simulink model "SdbRxSigProc"  (R2022b) (continued)
== system SdbRxSigProc == (continued)
  MemWrForFuTInFuHeatrMdl_MemWrForFuTInFuHeatrMdl : Outport  Port=12  OutDataTypeStr=Bus: MemRecForFuTInFuHeatrMdl  PortDimensions=1  BusOutputAsStruct=on
  MemWrForHeatgInpMgr_MemWrForHeatgInpMgr : Outport  Port=13  OutDataTypeStr=Bus: MemRecForHeatgInpMgr  PortDimensions=1  BusOutputAsStruct=on
  MemWrForSnsrMdlCooltT_MemWrForSnsrMdlCooltT : Outport  Port=14  OutDataTypeStr=Bus: MemRecForSnsrMdlCooltT  PortDimensions=1  BusOutputAsStruct=on
  SunData_SunData : Outport  Port=15  OutDataTypeStr=Bus: SunData  PortDimensions=1  BusOutputAsStruct=on
  SunLoadOutOfVeh_SunLoadOutOfVeh : Outport  Port=16  OutDataTypeStr=Bus: SunLoadOutOfVeh  PortDimensions=1  BusOutputAsStruct=on
  AACAirClngErrStsNgcc_AACAirClngErrStsNgcc : Outport  Port=17  OutDataTypeStr=Enum: AACAirClngErrSts  PortDimensions=1
  AACAirClngStsNgcc_AACAirClngStsNgcc : Outport  Port=18  OutDataTypeStr=Enum: AACAirClngSts  PortDimensions=1
  AFUFragraCh1AvlTiPrecNgcc_AFUFragraCh1AvlTiPrecNgcc : Outport  Port=19  OutMin=0  OutMax=100  OutDataTypeStr=Perc9  PortDimensions=1
  AFUFragraCh2AvlTiPrecNgcc_AFUFragraCh2AvlTiPrecNgcc : Outport  Port=20  OutMin=0  OutMax=100  OutDataTypeStr=Perc9  PortDimensions=1
  AFUFragraCh3AvlTiPrecNgcc_AFUFragraCh3AvlTiPrecNgcc : Outport  Port=21  OutMin=0  OutMax=100  OutDataTypeStr=Perc9  PortDimensions=1
  AFUFragraCh4AvlTiPrecNgcc_AFUFragraCh4AvlTiPrecNgcc : Outport  Port=22  OutMin=0  OutMax=100  OutDataTypeStr=Perc9  PortDimensions=1
  AFUFragraCh5AvlTiPrecNgcc_AFUFragraCh5AvlTiPrecNgcc : Outport  Port=23  OutMin=0  OutMax=100  OutDataTypeStr=Perc9  PortDimensions=1
  AirClngReq_AirClngReq : Outport  Port=24  OutDataTypeStr=Enum: OnOffNoReq  PortDimensions=1
  AirFragCh1OvrdDyNgcc_AirFragCh1OvrdDyNgcc : Outport  Port=25  OutDataTypeStr=Bus: DateTi30  PortDimensions=1  BusOutputAsStruct=on
  AirFragCh2OvrdDyNgcc_AirFragCh2OvrdDyNgcc : Outport  Port=26  OutDataTypeStr=Bus: DateTi30  PortDimensions=1  BusOutputAsStruct=on
  AirFragCh3OvrdDyNgcc_AirFragCh3OvrdDyNgcc : Outport  Port=27  OutDataTypeStr=Bus: DateTi30  PortDimensions=1  BusOutputAsStruct=on
  AirFragCh4OvrdDyNgcc_AirFragCh4OvrdDyNgcc : Outport  Port=28  OutDataTypeStr=Bus: DateTi30  PortDimensions=1  BusOutputAsStruct=on
  AirFragCh5OvrdDyNgcc_AirFragCh5OvrdDyNgcc : Outport  Port=29  OutDataTypeStr=Bus: DateTi30  PortDimensions=1  BusOutputAsStruct=on
  AirPtcEnadNgcc_AirPtcEnadNgcc : Outport  Port=30  OutDataTypeStr=Boolean  PortDimensions=1
  AirPtcHVPwrActlFirstLeNgcc_AirPtcHVPwrActlFirstLeNgcc : Outport  Port=31  OutMin=0  OutMax=20460  OutDataTypeStr=Pwr3  PortDimensions=1
  AirPtcHVPwrActlFirstRiNgcc_AirPtcHVPwrActlFirstRiNgcc : Outport  Port=32  OutMin=0  OutMax=20460  OutDataTypeStr=Pwr3  PortDimensions=1
  AirPtcHVPwrActlSecLeNgcc_AirPtcHVPwrActlSecLeNgcc : Outport  Port=33  OutMin=0  OutMax=20460  OutDataTypeStr=Pwr3  PortDimensions=1
  AirPtcModeReqNgcc_AirPtcModeReqNgcc : Outport  Port=34  OutDataTypeStr=Enum: AirPtcModeReq  PortDimensions=1
  AirSrcStsFbNgcc_AirSrcStsFbNgcc : Outport  Port=35  OutMin=0  OutMax=3  OutDataTypeStr=UInt2  PortDimensions=1
  AltitudeInfromNgcc_AltitudeInfromNgcc : Outport  Port=36  OutDataTypeStr=UInt15  PortDimensions=1
  AMBStsNgcc_AMBStsNgcc : Outport  Port=37  OutDataTypeStr=Bus: AMBStsgrp  PortDimensions=1  BusOutputAsStruct=on
  BackflushVlvErrNgcc_BackflushVlvErrNgcc : Outport  Port=38  OutMin=0  OutMax=3  OutDataTypeStr=UInt2  PortDimensions=1
  BookTrvlClimaActvReq_Ngcc_BookTrvlClimaActvReq : Outport  Port=39  OutDataTypeStr=Enum: OnOffNoReq  PortDimensions=1
  BusCurrentFbNgcc_BusCurrentFbNgcc : Outport  Port=40  OutDataTypeStr=PackCurrent  PortDimensions=1
  ChgFilElmWarnNgcc_ChgFilElmWarnNgcc : Outport  Port=41  OutMin=0  OutMax=3  OutDataTypeStr=UInt2  PortDimensions=1
  CmptmtOxygenConcQFNgcc_CmptmtOxygenConcQFNgcc : Outport  Port=42  OutDataTypeStr=Enum: Qf1  PortDimensions=1
  CmptmtOxygenConcVluNgcc_CmptmtOxygenConcVluNgcc : Outport  Port=43  OutMin=0  OutMax=100  OutDataTypeStr=Perc9  PortDimensions=1
  CoolgFanErrNgcc_CoolgFanErrNgcc : Outport  Port=44  OutMin=0  OutMax=3  OutDataTypeStr=UInt2  PortDimensions=1
  CourseDirFromNavNgcc_CourseDirFromNavNgcc : Outport  Port=45  OutDataTypeStr=Bus: NavDir  PortDimensions=1  BusOutputAsStruct=on
  CurrentCongestionInformationNgcc_CurrentCongestionInformationNgcc : Outport  Port=46  OutMin=0  OutMax=15  OutDataTypeStr=UInt4  PortDimensions=1
  CurrentTunnelInformationNgcc_CurrentTunnelInformationNgcc : Outport  Port=47  OutDataTypeStr=Enum: OnOffNoReq  PortDimensions=1
  DcDcActvdNgcc_DcDcActvdNgcc : Outport  Port=48  OutDataTypeStr=Enum: DcDcActvd  PortDimensions=1
  DcDcPwrLimNgcc_DcDcPwrLimNgcc : Outport  Port=49  OutDataTypeStr=Boolean  PortDimensions=1
  DHUResdSig10Ngcc_DHUResdSig10Ngcc : Outport  Port=50  OutDataTypeStr=UInt32  PortDimensions=1
  DHUResdSig11Ngcc_DHUResdSig11Ngcc : Outport  Port=51  OutDataTypeStr=Unit64  PortDimensions=1
  DHUResdSig12Ngcc_DHUResdSig12Ngcc : Outport  Port=52  OutDataTypeStr=Unit64  PortDimensions=1
  DHUResdSig13Ngcc_DHUResdSig13Ngcc : Outport  Port=53  OutDataTypeStr=UInt32  PortDimensions=1
  DHUResdSig3Ngcc_DHUResdSig3Ngcc : Outport  Port=54  OutDataTypeStr=UInt16  PortDimensions=1
  DHUResdSig4Ngcc_DHUResdSig4Ngcc : Outport  Port=55  OutDataTypeStr=UInt16  PortDimensions=1
  DHUResdSig5Ngcc_DHUResdSig5Ngcc : Outport  Port=56  OutDataTypeStr=UInt32  PortDimensions=1
  DHUResdSig6Ngcc_DHUResdSig6Ngcc : Outport  Port=57  OutDataTypeStr=Unit64  PortDimensions=1
  DHUResdSig7Ngcc_DHUResdSig7Ngcc : Outport  Port=58  OutDataTypeStr=Unit64  PortDimensions=1
  DHUResdSig8Ngcc_DHUResdSig8Ngcc : Outport  Port=59  OutDataTypeStr=UInt16  PortDimensions=1
  DHUResdSig9Ngcc_DHUResdSig9Ngcc : Outport  Port=60  OutDataTypeStr=UInt16  PortDimensions=1
  DispHvBattLvlOfChrgNgcc_DispHvBattLvlOfChrgNgcc : Outport  Port=61  OutMin=0  OutMax=102.30000000000001  OutDataTypeStr=EcoDrvInfo  PortDimensions=1
  DropTopPosnStsNgcc_DropTopPosnStsNgcc : Outport  Port=62  OutDataTypeStr=Enum: PosnSts  PortDimensions=1
  DrvrSeatPosPercNgcc_DrvrSeatPosPercNgcc : Outport  Port=63  OutDataTypeStr=Bus: SeatPosPercQF  PortDimensions=1  BusOutputAsStruct=on
  DrvrSeatSts_Ngcc_DrvrSeatSts : Outport  Port=64  OutDataTypeStr=Enum: OccptPresSt1  PortDimensions=1
  DrvrSeatVentnLvlSts2Ngcc_DrvrSeatVentnLvlSts2Ngcc : Outport  Port=65  OutDataTypeStr=Enum: SeatClimaLvl  PortDimensions=1
  FctvStsNgcc_FctvStsNgcc : Outport  Port=66  OutDataTypeStr=Bus: ValveSts  PortDimensions=1  BusOutputAsStruct=on
  FloatDrvingActvNgcc_FloatDrvingActvNgcc : Outport  Port=67  OutDataTypeStr=Bus: FloatDrvingActv  PortDimensions=1  BusOutputAsStruct=on
  HighTempCleanHmiReqNgcc_HighTempCleanHmiReqNgcc : Outport  Port=68  OutDataTypeStr=Enum: NoReqOnOff  PortDimensions=1
  HMIAirCdnrAutDrySetNgcc_HMIAirCdnrAutDrySetNgcc : Outport  Port=69  OutDataTypeStr=Enum: OnOff1  PortDimensions=1
  HmiAirDistbnRow1LeNgcc_HmiAirDistbnRow1LeNgcc : Outport  Port=70  OutDataTypeStr=Enum: HmiCmptmtAirDistbnFrnt  PortDimensions=1
  HmiAirDistbnRow1RiNgcc_HmiAirDistbnRow1RiNgcc : Outport  Port=71  OutDataTypeStr=Enum: HmiCmptmtAirDistbnFrnt  PortDimensions=1
  HmiAutoRow1LeNgcc_HmiAutoRow1LeNgcc : Outport  Port=72  OutDataTypeStr=Enum: OnOff1  PortDimensions=1
  HmiAutoRow1RiNgcc_HmiAutoRow1RiNgcc : Outport  Port=73  OutDataTypeStr=Enum: OnOff1  PortDimensions=1
  HmiAutOxySetNgcc_HmiAutOxySetNgcc : Outport  Port=74  OutDataTypeStr=Enum: OnOff1  PortDimensions=1
  HmiCarLockACRecircSetNgcc_HmiCarLockACRecircSetNgcc : Outport  Port=75  OutMin=0  OutMax=3  OutDataTypeStr=UInt2  PortDimensions=1
  HmiClimaRow1LePwrNgcc_HmiClimaRow1LePwrNgcc : Outport  Port=76  OutDataTypeStr=Enum: OnOff1  PortDimensions=1
  HmiClimaRow1PwrNgcc_HmiClimaRow1PwrNgcc : Outport  Port=77  OutDataTypeStr=Enum: OnOff1  PortDimensions=1
  HmiClimaRow1RiPwrNgcc_HmiClimaRow1RiPwrNgcc : Outport  Port=78  OutDataTypeStr=Enum: OnOff1  PortDimensions=1
  HmiClimaRow2PwrNgcc_HmiClimaRow2PwrNgcc : Outport  Port=79  OutDataTypeStr=Enum: OnOff1  PortDimensions=1
  HmiClimaRow3PwrNgcc_HmiClimaRow3PwrNgcc : Outport  Port=80  OutDataTypeStr=Enum: OnOff1  PortDimensions=1
  HMIClimaVentSetNgcc_HMIClimaVentSetNgcc : Outport  Port=81  OutDataTypeStr=Enum: OnOff1  PortDimensions=1
  HmiDrvrHeadPosnNgcc_HmiDrvrHeadPosnNgcc : Outport  Port=82  OutDataTypeStr=Bus: GazeDirOri  PortDimensions=1  BusOutputAsStruct=on
  HmiElecAirBodyVentLebtnNgcc_HmiElecAirBodyVentLebtnNgcc : Outport  Port=83  OutMin=0  OutMax=15  OutDataTypeStr=UInt4  PortDimensions=1
  HmiElecAirBodyVentRibtnNgcc_HmiElecAirBodyVentRibtnNgcc : Outport  Port=84  OutMin=0  OutMax=15  OutDataTypeStr=UInt4  PortDimensions=1
  HmiElecAirConsolePosnNgcc_HmiElecAirConsolePosnNgcc : Outport  Port=85  OutDataTypeStr=Bus: PosIndct  PortDimensions=1  BusOutputAsStruct=on
  HmiElecAirConsoleSwtLeNgcc_HmiElecAirConsoleSwtLeNgcc : Outport  Port=86  OutDataTypeStr=Enum: OnOff1  PortDimensions=1
  HmiElecAirConsoleSwtRiNgcc_HmiElecAirConsoleSwtRiNgcc : Outport  Port=87  OutDataTypeStr=Enum: OnOff1  PortDimensions=1
  HmiElecAirDirModeSecRowReqNgcc_HmiElecAirDirModeSecRowReqNgcc : Outport  Port=88  OutDataTypeStr=Bus: DirModReq1  PortDimensions=1  BusOutputAsStruct=on
  HmiElecAirDirSwngSecLeNgcc_HmiElecAirDirSwngSecLeNgcc : Outport  Port=89  OutDataTypeStr=Enum: OnOff1  PortDimensions=1
  HmiElecAirDirSwngSecRiNgcc_HmiElecAirDirSwngSecRiNgcc : Outport  Port=90  OutDataTypeStr=Enum: OnOff1  PortDimensions=1
  HmiElecAirDrvrNatureWindReqNgcc_HmiElecAirDrvrNatureWindReqNgcc : Outport  Port=91  OutDataTypeStr=Enum: OnOff1  PortDimensions=1
  HmiElecAirPassNatureWindReqNgcc_HmiElecAirPassNatureWindReqNgcc : Outport  Port=92  OutDataTypeStr=Enum: OnOff1  PortDimensions=1
  HMIFACSwtNgcc_HMIFACSwtNgcc : Outport  Port=93  OutDataTypeStr=Enum: OnOff1  PortDimensions=1
  HmiFanLvlRow1LeNgcc_HmiFanLvlRow1LeNgcc : Outport  Port=94  OutDataTypeStr=Enum: HmiHvacFanLvl  PortDimensions=1
  HmiFanLvlRow1RiNgcc_HmiFanLvlRow1RiNgcc : Outport  Port=95  OutDataTypeStr=Enum: HmiHvacFanLvl  PortDimensions=1
  HmiFragraChRatReq_HmiFragraChRatReq : Outport  Port=96  OutDataTypeStr=Bus: HmiFragraTypRatReq  PortDimensions=1  BusOutputAsStruct=on
  HmiFragraLvlReq_HmiFragraLvlReq : Outport  Port=97  OutDataTypeStr=Enum: HmiFragraLvlReq  PortDimensions=1
  HMILBRACStrtSetNgcc_HMILBRACStrtSetNgcc : Outport  Port=98  OutDataTypeStr=Enum: OnOff1  PortDimensions=1
  HmiOxyinCarSwtNgcc_HmiOxyinCarSwtNgcc : Outport  Port=99  OutDataTypeStr=Enum: NoReqOnOff  PortDimensions=1
  HmiParkVentSetNgcc_HmiParkVentSetNgcc : Outport  Port=100  OutDataTypeStr=Enum: OnOff1  PortDimensions=1
  HmiParkVentTmrSetNgcc_HmiParkVentTmrSetNgcc : Outport  Port=101  OutMin=0  OutMax=31  OutDataTypeStr=TimeHr5  PortDimensions=1
  HmiPassHeadPosnNgcc_HmiPassHeadPosnNgcc : Outport  Port=102  OutDataTypeStr=Bus: GazeDirOri  PortDimensions=1  BusOutputAsStruct=on
  HmiPopUpResp_HmiPopUpResp : Outport  Port=103  OutDataTypeStr=Enum: BookChargeSetResponse  PortDimensions=1
  HmiSmrtWindFolwSetNgcc_HmiSmrtWindFolwSetNgcc : Outport  Port=104  OutDataTypeStr=Enum: OnOff1  PortDimensions=1
  HMIThermoTankDoorOpenNgcc_HMIThermoTankDoorOpenNgcc : Outport  Port=105  OutDataTypeStr=Enum: OnOffNoReq  PortDimensions=1
  HMIThermoTankEcoSwNgcc_HMIThermoTankEcoSwNgcc : Outport  Port=106  OutDataTypeStr=Enum: NoReqOnOff  PortDimensions=1
  HMIThermoTankTNgcc_HMIThermoTankTNgcc : Outport  Port=107  OutDataTypeStr=TempAtTime  PortDimensions=1
  HmiThermoTSteDeoNewSwtNgcc_HmiThermoTSteDeoNewSwtNgcc : Outport  Port=108  OutDataTypeStr=UInt8  PortDimensions=1
  HmiThermoTSteDeoSwtNgcc_HmiThermoTSteDeoSwtNgcc : Outport  Port=109  OutDataTypeStr=Enum: OnOff1  PortDimensions=1
  HmiUVSterilSetNgcc_HmiUVSterilSetNgcc : Outport  Port=110  OutDataTypeStr=Enum: OnOff1  PortDimensions=1
  HmiWinterLockCarPrevnIceSetNgcc_HmiWinterLockCarPrevnIceSetNgcc : Outport  Port=111  OutDataTypeStr=Enum: OnOff1  PortDimensions=1
  HoodStsNgcc_HoodStsNgcc : Outport  Port=112  OutDataTypeStr=Enum: DoorSts2  PortDimensions=1
  HvBattLimnIndcnNgcc_HvBattLimnIndcnNgcc : Outport  Port=113  OutDataTypeStr=UInt16  PortDimensions=1
  InflateAirSrcSwtNgcc_InflateAirSrcSwtNgcc : Outport  Port=114  OutDataTypeStr=Enum: OnOffNoReq  PortDimensions=1
  InpVoltFbNgcc_InpVoltFbNgcc : Outport  Port=115  OutMin=0  OutMax=1638.3000000000002  OutDataTypeStr=RlyMeaPointVolt  PortDimensions=1
  LiOprnModNgcc_LiOprnModNgcc : Outport  Port=116  OutDataTypeStr=Enum: LiOperMod  PortDimensions=1
  LonglifeBatteryReqNgcc_LonglifeBatteryReqNgcc : Outport  Port=117  OutDataTypeStr=Enum: BatteryReq  PortDimensions=1
  McuOvrTempErrNgcc_McuOvrTempErrNgcc : Outport  Port=118  OutMin=0  OutMax=3  OutDataTypeStr=UInt2  PortDimensions=1
  McuTempFbNgcc_McuTempFbNgcc : Outport  Port=119  OutMin=-256  OutMax=255.9  OutDataTypeStr=MvBattTemp  PortDimensions=1
  McuTempSnsrErrNgcc_McuTempSnsrErrNgcc : Outport  Port=120  OutMin=0  OutMax=3  OutDataTypeStr=UInt2  PortDimensions=1
  MoleculeVlv1Ngcc_MoleculeVlv1Ngcc : Outport  Port=121  OutMin=0  OutMax=3  OutDataTypeStr=UInt2  PortDimensions=1
  MoleculeVlv2Ngcc_MoleculeVlv2Ngcc : Outport  Port=122  OutMin=0  OutMax=3  OutDataTypeStr=UInt2  PortDimensions=1
  MosOvrTempErrNgcc_MosOvrTempErrNgcc : Outport  Port=123  OutMin=0  OutMax=3  OutDataTypeStr=UInt2  PortDimensions=1
  MosTempFbNgcc_MosTempFbNgcc : Outport  Port=124  OutMin=-256  OutMax=255.9  OutDataTypeStr=MvBattTemp  PortDimensions=1
  MosTempSnsrErrNgcc_MosTempSnsrErrNgcc : Outport  Port=125  OutMin=0  OutMax=3  OutDataTypeStr=UInt2  PortDimensions=1
  MotStsFrmOGMNgcc_MotStsFrmOGMNgcc : Outport  Port=126  OutDataTypeStr=UInt8  PortDimensions=1
  MotTempFbNgcc_MotTempFbNgcc : Outport  Port=127  OutMin=-256  OutMax=255.9  OutDataTypeStr=MvBattTemp  PortDimensions=1
  MotTempSnsrErrNgcc_MotTempSnsrErrNgcc : Outport  Port=128  OutMin=0  OutMax=3  OutDataTypeStr=UInt2  PortDimensions=1
  OGMCmprSpdFbNgcc_OGMCmprSpdFbNgcc : Outport  Port=129  OutDataTypeStr=UInt16  PortDimensions=1
  OGMFlowFbNgcc_OGMFlowFbNgcc : Outport  Port=130  OutMin=0  OutMax=51.1  OutDataTypeStr=CooltFlow2  PortDimensions=1
  OGMHwVersNgcc_OGMHwVersNgcc : Outport  Port=131  OutDataTypeStr=UInt16  PortDimensions=1
  OGMIntErrNgcc_OGMIntErrNgcc : Outport  Port=132  OutDataTypeStr=Enum: Err1  PortDimensions=1
  OGMOvrVoltRangErrNgcc_OGMOvrVoltRangErrNgcc : Outport  Port=133  OutDataTypeStr=Enum: Err1  PortDimensions=1
  OGMRunTiNgcc_OGMRunTiNgcc : Outport  Port=134  OutDataTypeStr=UInt32  PortDimensions=1
  OGMSwVersNgcc_OGMSwVersNgcc : Outport  Port=135  OutDataTypeStr=UInt16  PortDimensions=1
  OGMTempErrNgcc_OGMTempErrNgcc : Outport  Port=136  OutDataTypeStr=Enum: TempErr  PortDimensions=1
  OGMUndVoltRangErrNgcc_OGMUndVoltRangErrNgcc : Outport  Port=137  OutDataTypeStr=Enum: Err1  PortDimensions=1
  OxygenConcFbFrmOGMNgcc_OxygenConcFbFrmOGMNgcc : Outport  Port=138  OutMin=0  OutMax=100  OutDataTypeStr=Perc9  PortDimensions=1
  OxygenConcFltNgcc_OxygenConcFltNgcc : Outport  Port=139  OutMin=0  OutMax=3  OutDataTypeStr=UInt2  PortDimensions=1
  OxygenConcSnsrElecErrNgcc_OxygenConcSnsrElecErrNgcc : Outport  Port=140  OutDataTypeStr=Enum: OxygenConcSnsrElecErr  PortDimensions=1
  OxygenConcSnsrIntErrNgcc_OxygenConcSnsrIntErrNgcc : Outport  Port=141  OutDataTypeStr=Enum: Err1  PortDimensions=1
  OxygenConcSnsrOvrVoltRangErrNgcc_OxygenConcSnsrOvrVoltRangErrNgcc : Outport  Port=142  OutDataTypeStr=Enum: Err1  PortDimensions=1
  OxygenConcSnsrStsFrmOGMNgcc_OxygenConcSnsrStsFrmOGMNgcc : Outport  Port=143  OutMin=0  OutMax=3  OutDataTypeStr=UInt2  PortDimensions=1
  OxygenConcSnsrStsNgcc_OxygenConcSnsrStsNgcc : Outport  Port=144  OutDataTypeStr=Enum: PmSnsrSts  PortDimensions=1
  OxygenConcSnsrTempErrNgcc_OxygenConcSnsrTempErrNgcc : Outport  Port=145  OutDataTypeStr=Enum: TempErr  PortDimensions=1
  OxygenConcSnsrUndVoltRangErrNgcc_OxygenConcSnsrUndVoltRangErrNgcc : Outport  Port=146  OutDataTypeStr=Enum: Err1  PortDimensions=1
  OxygenGenrErrInfoNgcc_OxygenGenrErrInfoNgcc : Outport  Port=147  OutDataTypeStr=Enum: OxygengenrErrInfo  PortDimensions=1
  OxygenSplyAvlStsNgcc_OxygenSplyAvlStsNgcc : Outport  Port=148  OutDataTypeStr=Enum: OxygenSplyAvlSts  PortDimensions=1
  OxygenSplyErrNgcc_OxygenSplyErrNgcc : Outport  Port=149  OutDataTypeStr=Enum: Err1  PortDimensions=1
  OxygenTubeBlkNgcc_OxygenTubeBlkNgcc : Outport  Port=150  OutMin=0  OutMax=3  OutDataTypeStr=UInt2  PortDimensions=1
  PassSeatPosPercNgcc_PassSeatPosPercNgcc : Outport  Port=151  OutDataTypeStr=Bus: SeatPosPercQF  PortDimensions=1  BusOutputAsStruct=on
  PassSeatSts2_Ngcc_PassSeatSts2 : Outport  Port=152  OutDataTypeStr=Enum: OccptPresSt1  PortDimensions=1
  PassSeatVentnLvlSts2Ngcc_PassSeatVentnLvlSts2Ngcc : Outport  Port=153  OutDataTypeStr=Enum: SeatClimaLvl  PortDimensions=1
  PhaseCurrentFbNgcc_PhaseCurrentFbNgcc : Outport  Port=154  OutDataTypeStr=PackCurrent  PortDimensions=1
  PosnBriefNgcc_PosnBriefNgcc : Outport  Port=155  OutDataTypeStr=Bus: Posn  PortDimensions=1  BusOutputAsStruct=on
  PressureFbNgcc_PressureFbNgcc : Outport  Port=156  OutMin=0  OutMax=400  OutDataTypeStr=Pressure2  PortDimensions=1
  PressureFltNgcc_PressureFltNgcc : Outport  Port=157  OutMin=0  OutMax=3  OutDataTypeStr=UInt2  PortDimensions=1
  PressureSnsrErrNgcc_PressureSnsrErrNgcc : Outport  Port=158  OutMin=0  OutMax=3  OutDataTypeStr=UInt2  PortDimensions=1
  PwrCnsForHvacHeatrElecRe_PwrCnsForHvacHeatrElecRe : Outport  Port=159  OutDataTypeStr=Pwr33  PortDimensions=1
  RefrLpSwStsNgcc_RefrLpSwStsNgcc : Outport  Port=160  OutDataTypeStr=Enum: CloseOpen  PortDimensions=1
  RefrtorVlvEnaNgcc_RefrtorVlvEnaNgcc : Outport  Port=161  OutDataTypeStr=Enum: OnOff1  PortDimensions=1
  RoadInclnNgcc_RoadInclnNgcc : Outport  Port=162  OutDataTypeStr=Bus: RoadInclnRec1  PortDimensions=1  BusOutputAsStruct=on
  RuranACModeSetNgcc_RuranACModeSetNgcc : Outport  Port=163  OutDataTypeStr=Enum: RuranACModeSet  PortDimensions=1
  SceneModSeld_Ngcc_SceneModSeld : Outport  Port=164  OutDataTypeStr=Enum: PatSeld  PortDimensions=1
  SeatBackAngleRowFirstDrvrNgcc_SeatBackAngleRowFirstDrvrNgcc : Outport  Port=165  OutMin=0  OutMax=180  OutDataTypeStr=AngleDeg180  PortDimensions=1
  SeatBackAngleRowFirstPassNgcc_SeatBackAngleRowFirstPassNgcc : Outport  Port=166  OutMin=0  OutMax=180  OutDataTypeStr=AngleDeg180  PortDimensions=1
  SeatOccptAtRowSecDrSide2_Ngcc_SeatOccptAtRowSecDrSide2 : Outport  Port=167  OutDataTypeStr=Enum: OccptPresSt1  PortDimensions=1
  SeatOccptAtRowSecMid2_Ngcc_SeatOccptAtRowSecMid2 : Outport  Port=168  OutDataTypeStr=Enum: OccptPresSt1  PortDimensions=1
  SeatOccptAtRowSecPasSide2_Ngcc_SeatOccptAtRowSecPasSide2 : Outport  Port=169  OutDataTypeStr=Enum: OccptPresSt1  PortDimensions=1
  SeatOccptAtRowThrdLe2Ngcc_SeatOccptAtRowThrdLe2Ngcc : Outport  Port=170  OutDataTypeStr=Enum: OccptPresSt1  PortDimensions=1
  SeatOccptAtRowThrdRi2Ngcc_SeatOccptAtRowThrdRi2Ngcc : Outport  Port=171  OutDataTypeStr=Enum: OccptPresSt1  PortDimensions=1
  SportModeThmgtRequestNgcc_SportModeThmgtRequestNgcc : Outport  Port=172  OutDataTypeStr=Enum: ThmgtLevel  PortDimensions=1
  TCSCooltFlowNgcc_TCSCooltFlowNgcc : Outport  Port=173  OutDataTypeStr=CooltFlow1  PortDimensions=1
  TCSCooltTNgcc_TCSCooltTNgcc : Outport  Port=174  OutDataTypeStr=TempAtTime  PortDimensions=1
  TcsDoorEleBailReqNgcc_TcsDoorEleBailReqNgcc : Outport  Port=175  OutDataTypeStr=Enum: WhlMotSysCluReq  PortDimensions=1
  TcsFanReqNgcc_TcsFanReqNgcc : Outport  Port=176  OutMin=0  OutMax=15  OutDataTypeStr=UInt4  PortDimensions=1
  TcsModSwtReqNgcc_TcsModSwtReqNgcc : Outport  Port=177  OutDataTypeStr=Enum: TelmThermoTankReq  PortDimensions=1
  TcsOvrheatdProtnStsNgcc_TcsOvrheatdProtnStsNgcc : Outport  Port=178  OutMin=0  OutMax=3  OutDataTypeStr=UInt2  PortDimensions=1
  TcsSemiconReqNgcc_TcsSemiconReqNgcc : Outport  Port=179  OutMin=0  OutMax=15  OutDataTypeStr=UInt4  PortDimensions=1
  TcsTmrOverReqNgcc_TcsTmrOverReqNgcc : Outport  Port=180  OutMin=0  OutMax=31  OutDataTypeStr=TimeHr5  PortDimensions=1
  TcsTNowCtrlReqNgcc_TcsTNowCtrlReqNgcc : Outport  Port=181  OutDataTypeStr=TempAtTime  PortDimensions=1
  TcsTopDoorEleBailReqNgcc_TcsTopDoorEleBailReqNgcc : Outport  Port=182  OutDataTypeStr=Enum: WhlMotSysCluReq  PortDimensions=1
  TelmOxygenValReqNgcc_TelmOxygenValReqNgcc : Outport  Port=183  OutDataTypeStr=Enum: OnOffNoReq  PortDimensions=1
  TelmThermoTankECOSetNgcc_TelmThermoTankECOSetNgcc : Outport  Port=184  OutDataTypeStr=Enum: NoReqOnOff  PortDimensions=1
  TelmThermoTankTNgcc_TelmThermoTankTNgcc : Outport  Port=185  OutDataTypeStr=EngT2  PortDimensions=1
  ThermHvacRecircReqEnaNgcc_ThermHvacRecircReqEnaNgcc : Outport  Port=186  OutDataTypeStr=Boolean  PortDimensions=1
  ThermHvacRecircReqNgcc_ThermHvacRecircReqNgcc : Outport  Port=187  OutMin=0  OutMax=100  OutDataTypeStr=Perc6  PortDimensions=1
  ThrdRowSeatHeatgActlPwrCnsLeNgcc_ThrdRowSeatHeatgActlPwrCnsLeNgcc : Outport  Port=188  OutDataTypeStr=UInt8  PortDimensions=1
  ThrdRowSeatHeatgActlPwrCnsRiNgcc_ThrdRowSeatHeatgActlPwrCnsRiNgcc : Outport  Port=189  OutDataTypeStr=UInt8  PortDimensions=1
  ThrdRowSeatHeatgDesPwrCnsLeNgcc_ThrdRowSeatHeatgDesPwrCnsLeNgcc : Outport  Port=190  OutDataTypeStr=UInt8  PortDimensions=1
  ThrdRowSeatHeatgDesPwrCnsRiNgcc_ThrdRowSeatHeatgDesPwrCnsRiNgcc : Outport  Port=191  OutDataTypeStr=UInt8  PortDimensions=1
  ThrdRowSeatVentnActlPwrCnsLeNgcc_ThrdRowSeatVentnActlPwrCnsLeNgcc : Outport  Port=192  OutDataTypeStr=UInt8  PortDimensions=1
  ThrdRowSeatVentnActlPwrCnsRiNgcc_ThrdRowSeatVentnActlPwrCnsRiNgcc : Outport  Port=193  OutDataTypeStr=UInt8  PortDimensions=1
  ThrdRowSeatVentnDesPwrCnsLeNgcc_ThrdRowSeatVentnDesPwrCnsLeNgcc : Outport  Port=194  OutDataTypeStr=UInt8  PortDimensions=1
  ThrdRowSeatVentnDesPwrCnsRiNgcc_ThrdRowSeatVentnDesPwrCnsRiNgcc : Outport  Port=195  OutDataTypeStr=UInt8  PortDimensions=1
  UseEvapReqTMinNewNgcc_UseEvapReqTMinNewNgcc : Outport  Port=196  OutDataTypeStr=Enum: OnOff1  PortDimensions=1
  VehTiAndDataNgcc_VehTiAndDataNgcc : Outport  Port=197  OutDataTypeStr=Bus: DateTi30  PortDimensions=1  BusOutputAsStruct=on
  VlvVoltFltNgcc_VlvVoltFltNgcc : Outport  Port=198  OutMin=0  OutMax=3  OutDataTypeStr=UInt2  PortDimensions=1
  WarnLvlMsgNgcc_WarnLvlMsgNgcc : Outport  Port=199  OutDataTypeStr=Enum: WarnLvl  PortDimensions=1
  WasRespLevelNgcc_WasRespLevelNgcc : Outport  Port=200  OutDataTypeStr=Enum: WasRespLevel  PortDimensions=1
  WaterDepthInformationNgcc_WaterDepthInformationNgcc : Outport  Port=201  OutDataTypeStr=Bus: WaterDepthInformation  PortDimensions=1  BusOutputAsStruct=on
  WeatherQualityAQINgcc_WeatherQualityAQINgcc : Outport  Port=202  OutMin=0  OutMax=1000  OutDataTypeStr=LineLenWithVehForBkp  PortDimensions=1
  WinPosnStsDrv_WinPosnStsAtDrv : Outport  Port=203  OutDataTypeStr=Enum: WinAndRoofAndCurtPosnTyp  PortDimensions=1
  ActvnOfWshrFrntSafe_Ngcc_ActvnOfWshrFrntSafe : Outport  Port=204  OutDataTypeStr=Enum: OnOffCrit1  PortDimensions=1
  AirMtrlSnsrInAirQlyIdx_AirMtrlSnsrInAirQlyIdx : Outport  Port=205  OutDataTypeStr=AirMtrlSnsrAirQlyIdx  PortDimensions=1
  AirTAftHeatElmEstimd_AirTAftHeatElmEstimd : Outport  Port=206  OutDataTypeStr=T30  PortDimensions=1
  AirTFromHeatgEstimd_Ngcc_AirTFromHeatgEstimd : Outport  Port=207  OutDataTypeStr=T30  PortDimensions=1
  AsyFanSts_AsyFanSts : Outport  Port=208  OutDataTypeStr=Enum: OnOff1  PortDimensions=1
  BltLockStAtDrvr_Ngcc_BltLockStAtDrvr : Outport  Port=209  OutDataTypeStr=Bus: BltLockStFrnt  PortDimensions=1  BusOutputAsStruct=on
  BltLockStAtPass_Ngcc_BltLockStAtPass : Outport  Port=210  OutDataTypeStr=Bus: BltLockStFrnt  PortDimensions=1  BusOutputAsStruct=on
  BltLockStAtRowSecLe_Ngcc_BltLockStAtRowSecLe : Outport  Port=211  OutDataTypeStr=Bus: BltLockStRe  PortDimensions=1  BusOutputAsStruct=on
  BltLockStAtRowSecMid_Ngcc_BltLockStAtRowSecMid : Outport  Port=212  OutDataTypeStr=Bus: BltLockStRe  PortDimensions=1  BusOutputAsStruct=on
  BltLockStAtRowSecRi_Ngcc_BltLockStAtRowSecRi : Outport  Port=213  OutDataTypeStr=Bus: BltLockStRe  PortDimensions=1  BusOutputAsStruct=on
  BltLockStAtRowThrdLe_Ngcc_BltLockStAtRowThrdLe : Outport  Port=214  OutDataTypeStr=Bus: BltLockStRe  PortDimensions=1  BusOutputAsStruct=on
  BltLockStAtRowThrdRi_Ngcc_BltLockStAtRowThrdRi : Outport  Port=215  OutDataTypeStr=Bus: BltLockStRe  PortDimensions=1  BusOutputAsStruct=on
  CamHeatrActv_CamHeatrActv : Outport  Port=216  OutDataTypeStr=Enum: OnOff1  PortDimensions=1
  CarTiGlb_Ngcc_CarTiGlb : Outport  Port=217  OutDataTypeStr=CarTiGlb  PortDimensions=1
  ClimaCmd_Ngcc_ClimaCmd : Outport  Port=218  OutDataTypeStr=Enum: OkNotOk  PortDimensions=1
  ClimaPwrAllwd_Ngcc_ClimaPwrAllwd : Outport  Port=219  OutMin=0  OutMax=2047  OutDataTypeStr=Pwr30  PortDimensions=1
  ClimaRqrd1ConnGen2_ClimaRqrd1ConnGen2 : Outport  Port=220  OutDataTypeStr=Enum: OnOffNoReq  PortDimensions=1
  ClimaRqrdFromRem1_ClimaRqrdFromRem1 : Outport  Port=221  OutDataTypeStr=Enum: OnOffNoReq  PortDimensions=1
  ClimaTmr_ClimaTmr : Outport  Port=222  OutDataTypeStr=CarTiGlb  PortDimensions=1
  ClimaTmrStsRqrd_ClimaTmrStsRqrd : Outport  Port=223  OutDataTypeStr=Enum: OnOff1  PortDimensions=1
  ClngRqrdFromHmi_ClngRqrdFromHmi : Outport  Port=224  OutDataTypeStr=Enum: OnOffNoReq  PortDimensions=1
  ClngRqrdFromTelm_ClngRqrdFromTelm : Outport  Port=225  OutDataTypeStr=Enum: OnOffNoReq  PortDimensions=1
  ClngRqrdFromTelmConnGen2_ClngRqrdFromTelmConnGen2 : Outport  Port=226  OutDataTypeStr=Enum: OnOffNoReq  PortDimensions=1
  CmpmtInAirPmAQI_Ngcc_CmpmtInAirPmAQI : Outport  Port=227  OutDataTypeStr=Enum: CmpmtAirPmLvl  PortDimensions=1
  CmpmtInAirPmDnsty_Ngcc_CmpmtInAirPmDnsty : Outport  Port=228  OutDataTypeStr=UInt10  PortDimensions=1
  CmptmtClngPreStrtReqNgcc_CmptmtClngPreStrtReq : Outport  Port=229  OutDataTypeStr=Enum: OnOffNoReq  PortDimensions=1
  CmptmtCoolgSts_Ngcc_CmptmtCoolgSts : Outport  Port=230  OutDataTypeStr=Enum: CmptmtCoolgSts  PortDimensions=1
  CoolgLoad_CoolgLoad : Outport  Port=231  OutDataTypeStr=Bus: CoolgLoad1  PortDimensions=1  BusOutputAsStruct=on
  CoolgReqForDispCen_CoolgReqForDispCen : Outport  Port=232  OutDataTypeStr=Enum: CoolgReqForDisp  PortDimensions=1
  CoolgReqForDrvrDisp_CoolgReqForDrvrDisp : Outport  Port=233  OutDataTypeStr=Enum: CoolgReqForDisp  PortDimensions=1
  CooltTForPrkgClima_CooltTForPrkgClima : Outport  Port=234  OutDataTypeStr=T30  PortDimensions=1
  CooltTInHeatElm_CooltTInHeatElm : Outport  Port=235  OutDataTypeStr=T30  PortDimensions=1
  CooltVlvClsd_CooltVlvClsd : Outport  Port=236  OutDataTypeStr=Boolean  PortDimensions=1
  CupHoldrDesPwr_CupHoldrDesPwr : Outport  Port=237  OutDataTypeStr=UInt7  PortDimensions=1
  DefrstSts_Ngcc_DefrstSts : Outport  Port=238  OutDataTypeStr=Bus: DefrstSts  PortDimensions=1  BusOutputAsStruct=on
  DoorDrvrReSts_DoorDrvrReSts : Outport  Port=239  OutDataTypeStr=Enum: DoorSts2  PortDimensions=1
  DoorDrvrSts_Ngcc_DoorDrvrSts : Outport  Port=240  OutDataTypeStr=Enum: DoorSts2  PortDimensions=1
  DoorPassReSts_DoorPassReSts : Outport  Port=241  OutDataTypeStr=Enum: DoorSts2  PortDimensions=1
  DoorPassSts_Ngcc_DoorPassSts : Outport  Port=242  OutDataTypeStr=Enum: DoorSts2  PortDimensions=1
  DrvModReq_Ngcc_DrvModReq : Outport  Port=243  OutDataTypeStr=Enum: DrvModReqType1  PortDimensions=1
  DrvrAirCdnrSetg_Ngcc_DrvrAirCdnrSetg : Outport  Port=244  OutDataTypeStr=Bus: DrvrAirCdnrSetg  PortDimensions=1  BusOutputAsStruct=on
  DrvrSeatHeatgActlPwrCns_Ngcc_DrvrSeatHeatgActlPwrCns : Outport  Port=245  OutDataTypeStr=UInt8  PortDimensions=1
  DrvrSeatHeatgDesPwrCns_Ngcc_DrvrSeatHeatgDesPwrCns : Outport  Port=246  OutDataTypeStr=UInt8  PortDimensions=1
  DrvrSeatVentnDesPwrCns_Ngcc_DrvrSeatVentnDesPwrCns : Outport  Port=247  OutDataTypeStr=UInt8  PortDimensions=1
  EcuKeepActvFromHeatg_EcuKeepActvFromHeatg : Outport  Port=248  OutDataTypeStr=Enum: OnOff1  PortDimensions=1
  EgyCnsAllwdForClima_Ngcc_EgyCnsAllwdForClima : Outport  Port=249  OutDataTypeStr=Egy2  PortDimensions=1
  EgySave_EgySave : Outport  Port=250  OutDataTypeStr=Enum: OnOff1  PortDimensions=1
  EngReqInHybForHeatg_EngReqInHybForHeatg : Outport  Port=251  OutDataTypeStr=Boolean  PortDimensions=1
  EngSt1WdSts_Ngcc_EngSt1WdSts : Outport  Port=252  OutDataTypeStr=Enum: EngSt1  PortDimensions=1
  HeatdCupPwrCnsAct_HeatdCupPwrCnsAct : Outport  Port=253  OutDataTypeStr=UInt7  PortDimensions=1
  HeatgPwrDmdEstimd_HeatgPwrDmdEstimd : Outport  Port=254  OutDataTypeStr=Pwr33  PortDimensions=1
  HeatrActvnReq_HeatrActvnReq : Outport  Port=255  OutDataTypeStr=Enum: OnOff1  PortDimensions=1
  HMIClimaEgySaveReq_Ngcc_HMIClimaEgySaveReq : Outport  Port=256  OutDataTypeStr=Enum: VentnActr01BlckInd  PortDimensions=1
  HmiClimaFrntAutReq_HmiClimaFrntAutReq : Outport  Port=257  OutDataTypeStr=Enum: OnOff1  PortDimensions=1
  HmiClimaReAutReq_HmiClimaReAutReq : Outport  Port=258  OutDataTypeStr=Enum: OnOff1  PortDimensions=1
  HmiCmptmtAirDistbnFrntLe_HmiCmptmtAirDistbnFrntLe : Outport  Port=259  OutDataTypeStr=Enum: HmiCmptmtAirDistbnFrnt  PortDimensions=1
  HmiCmptmtAirDistbnRe_HmiCmptmtAirDistbnRe : Outport  Port=260  OutDataTypeStr=Enum: HmiCmptmtAirDistbnFrnt  PortDimensions=1
  HmiCmptmtCoolgReq_HmiCmptmtCoolgReq : Outport  Port=261  OutDataTypeStr=Enum: HmiCmptmtCoolgReq  PortDimensions=1
  HmiDefrstMaxReq_HmiDefrstMaxReq : Outport  Port=262  OutDataTypeStr=Enum: ActrReq  PortDimensions=1
  HmiHvacFanLvlFrnt_HmiHvacFanLvlFrnt : Outport  Port=263  OutDataTypeStr=Enum: HmiHvacFanLvl  PortDimensions=1
  HmiHvacFanLvlRe_HmiHvacFanLvlRe : Outport  Port=264  OutDataTypeStr=Enum: HmiHvacFanLvl  PortDimensions=1
  HmiMaxACReq_HmiMaxACReq : Outport  Port=265  OutDataTypeStr=Enum: OnOff1  PortDimensions=1
  HmiPassTempSyncSet_HmiPassTempSyncSet : Outport  Port=266  OutDataTypeStr=Enum: OnOff1  PortDimensions=1
  HpcDeiceReq_Ngcc_HpcDeiceReq : Outport  Port=267  OutDataTypeStr=Enum: DeiceReq  PortDimensions=1
  HvacFanIFrnt_HvacFanIFrnt : Outport  Port=268  OutDataTypeStr=HvacFanI  PortDimensions=1
  HvacFanIRe_HvacFanIRe : Outport  Port=269  OutDataTypeStr=HvacFanI  PortDimensions=1
  HvacFanMotURe_HvacFanMotURe : Outport  Port=270  OutDataTypeStr=BattU  PortDimensions=1
  HvacFanSpdFrnt_HvacFanSpdFrnt : Outport  Port=271  OutDataTypeStr=HvacFanSpd  PortDimensions=1
  HvPwrEgyPrio_Ngcc_HvPwrEgyPrio : Outport  Port=272  OutDataTypeStr=Enum: HvPwrEgyPrio  PortDimensions=1
  IHUAPSts_Ngcc_IHUAPSts : Outport  Port=273  OutDataTypeStr=Enum: OnOff1  PortDimensions=1
  IncomingAirQlyCtrlFromHmi_Ngcc_IncomingAirQlyCtrlFromHmi : Outport  Port=274  OutDataTypeStr=Enum: OnOff1  PortDimensions=1
  IncomingAirQlyPopUpReq_Ngcc_IncomingAirQlyPopUpReq : Outport  Port=275  OutDataTypeStr=Enum: OnOff1  PortDimensions=1
  InPm25FanErr_Ngcc_InPm25FanErr : Outport  Port=276  OutDataTypeStr=Enum: FanErr  PortDimensions=1
  InPm25SnsrSts_Ngcc_InPm25SnsrSts : Outport  Port=277  OutDataTypeStr=Enum: PmSnsrSts  PortDimensions=1
  InteCleanUnpleSmell_Ngcc_InteCleanUnpleSmell : Outport  Port=278  OutDataTypeStr=Enum: OnOff1  PortDimensions=1
  LoadForCmprForAirCdn_LoadForCmprForAirCdn : Outport  Port=279  OutDataTypeStr=Perc4  PortDimensions=1
  LockgCenSts_Ngcc_LockgCenSts : Outport  Port=280  OutDataTypeStr=Bus: LockgCenSts3  PortDimensions=1  BusOutputAsStruct=on
  MemSaveFlgForCooltHeatrActvnCtrl_MemSaveFlgForCooltHeatrActvnCtrl : Outport  Port=281  OutDataTypeStr=Boolean  PortDimensions=1
  MemSaveFlgForCooltHeatrCtrl_MemSaveFlgForCooltHeatrCtrl : Outport  Port=282  OutDataTypeStr=Boolean  PortDimensions=1
  MemSaveFlgForFuTInHeatrMdl_MemSaveFlgForFuTInHeatrMdl : Outport  Port=283  OutDataTypeStr=Boolean  PortDimensions=1
  MemSaveFlgForHeatgInpMgr_MemSaveFlgForHeatgInpMgr : Outport  Port=284  OutDataTypeStr=Boolean  PortDimensions=1
  MemSaveFlgForSnsrMdlCooltT_MemSaveFlgForSnsrMdlCooltT : Outport  Port=285  OutDataTypeStr=Boolean  PortDimensions=1
  NetReqForHeatg_NetReqForHeatg : Outport  Port=286  OutDataTypeStr=Boolean  PortDimensions=1
  OnBdChrgrHndlSts_OnBdChrgrHndlSts : Outport  Port=287  OutDataTypeStr=Enum: OnBdChrgrSts1  PortDimensions=1
  PassSeatHeatgActlPwrCns_Ngcc_PassSeatHeatgActlPwrCns : Outport  Port=288  OutDataTypeStr=UInt8  PortDimensions=1
  PassSeatHeatgDesPwrCns_Ngcc_PassSeatHeatgDesPwrCns : Outport  Port=289  OutDataTypeStr=UInt8  PortDimensions=1
  PassSeatVentnActlPwrCns_Ngcc_PassSeatVentnActlPwrCns : Outport  Port=290  OutDataTypeStr=UInt8  PortDimensions=1
  PassSeatVentnDesPwrCns_Ngcc_PassSeatVentnDesPwrCns : Outport  Port=291  OutDataTypeStr=UInt8  PortDimensions=1
  SplyUForClimaCtrl_SplyUForClimaCtrl : Outport  Port=292  OutDataTypeStr=BattU  PortDimensions=1
  PostDrvgClimaReq_Ngcc_PostDrvgClimaReq : Outport  Port=293  OutDataTypeStr=Enum: OnOff1  PortDimensions=1
  PrkgHeatgActv_PrkgHeatgActv : Outport  Port=294  OutDataTypeStr=Boolean  PortDimensions=1
  PrkgHeatrStrtd_PrkgHeatrStrtd : Outport  Port=295  OutDataTypeStr=Boolean  PortDimensions=1
  PwrCnsDesForSeatHeatg_PwrCnsDesForSeatHeatg : Outport  Port=296  OutDataTypeStr=Pwr33  PortDimensions=1
  PwrCnsDesForSeatVent_PwrCnsDesForSeatVent : Outport  Port=297  OutDataTypeStr=Pwr33  PortDimensions=1
  PwrCnsDurgEngStopForFuHeatr_PwrCnsDurgEngStopForFuHeatr : Outport  Port=298  OutDataTypeStr=Pwr33  PortDimensions=1
  PwrCnsDurgEngStopForHvacCooltVlv_PwrCnsDurgEngStopForHvacCooltVlv : Outport  Port=299  OutDataTypeStr=Pwr33  PortDimensions=1
  PwrCnsDurgEngStopForWtrPmpAux_PwrCnsDurgEngStopForWtrPmpAux : Outport  Port=300  OutDataTypeStr=Pwr33  PortDimensions=1
  PwrCnsForFuHeatr_PwrCnsForFuHeatr : Outport  Port=301  OutDataTypeStr=Pwr33  PortDimensions=1
  PwrCnsForHvacCooltVlv_PwrCnsForHvacCooltVlv : Outport  Port=302  OutDataTypeStr=Pwr33  PortDimensions=1
  PwrCnsForHvacHeatrElecFrnt_PwrCnsForHvacHeatrElecFrnt : Outport  Port=303  OutDataTypeStr=Pwr33  PortDimensions=1
  PwrCnsForSeatHeatg_PwrCnsForSeatHeatg : Outport  Port=304  OutDataTypeStr=Pwr33  PortDimensions=1
  PwrCnsForWtrPmpAux_PwrCnsForWtrPmpAux : Outport  Port=305  OutDataTypeStr=Pwr33  PortDimensions=1
  PwrStsForPrkgHeatrElec_PwrStsForPrkgHeatrElec : Outport  Port=306  OutDataTypeStr=Boolean  PortDimensions=1
  RainfallAmnt_Ngcc_RainfallAmnt : Outport  Port=307  OutDataTypeStr=Enum: AmntSnsr  PortDimensions=1
  RemStrtHvCtrlReq_Ngcc_RemStrtHvCtrlReq : Outport  Port=308  OutDataTypeStr=Bus: RemStrHvCtrlReq  PortDimensions=1  BusOutputAsStruct=on
  SeatHeatDurgClimaEnad_SeatHeatDurgClimaEnad : Outport  Port=309  OutDataTypeStr=Enum: SeatHeatDurgClimaEnad  PortDimensions=1
  SeatHeatDurgClimaEnadConnGen2_SeatHeatDurgClimaEnadConnGen2 : Outport  Port=310  OutDataTypeStr=Enum: SeatHeatDurgClimaEnad  PortDimensions=1
  SeatHeatDurgClimaEnadFromTelm_Ngcc_SeatHeatDurgClimaEnadFromTelm : Outport  Port=311  OutDataTypeStr=Enum: SeatHeatDurgClimaEnad  PortDimensions=1
  SecRowSeatHeatgActlPwrCnsLe_Ngcc_SecRowSeatHeatgActlPwrCnsLe : Outport  Port=312  OutDataTypeStr=UInt8  PortDimensions=1
  SecRowSeatHeatgActlPwrCnsRi_Ngcc_SecRowSeatHeatgActlPwrCnsRi : Outport  Port=313  OutDataTypeStr=UInt8  PortDimensions=1
  SecRowSeatHeatgDesPwrCnsLe_Ngcc_SecRowSeatHeatgDesPwrCnsLe : Outport  Port=314  OutDataTypeStr=UInt8  PortDimensions=1
  SecRowSeatHeatgDesPwrCnsRi_Ngcc_SecRowSeatHeatgDesPwrCnsRi : Outport  Port=315  OutDataTypeStr=UInt8  PortDimensions=1
  SecRowSeatVentnActlPwrCnsLe_Ngcc_SecRowSeatVentnActlPwrCnsLe : Outport  Port=316  OutDataTypeStr=UInt8  PortDimensions=1
  SecRowSeatVentnActlPwrCnsRi_Ngcc_SecRowSeatVentnActlPwrCnsRi : Outport  Port=317  OutDataTypeStr=UInt8  PortDimensions=1
  SecRowSeatVentnDesPwrCnsLe_Ngcc_SecRowSeatVentnDesPwrCnsLe : Outport  Port=318  OutDataTypeStr=UInt8  PortDimensions=1
  SecRowSeatVentnDesPwrCnsRi_Ngcc_SecRowSeatVentnDesPwrCnsRi : Outport  Port=319  OutDataTypeStr=UInt8  PortDimensions=1
  SessionActvOutOfVeh_SessionActvOutOfVeh : Outport  Port=320  OutDataTypeStr=Enum: NoYes1  PortDimensions=1
  SteerWhlHeatgDesPwr_Ngcc_SteerWhlHeatgDesPwr : Outport  Port=321  OutDataTypeStr=UInt8  PortDimensions=1
  SteerWhlHeatgDurgClimaEnad_SteerWhlHeatgDurgClimaEnad : Outport  Port=322  OutDataTypeStr=Enum: OnOff1  PortDimensions=1
  SteerWhlHeatgDurgClimEandFromTelmConnGen2_SteerWhlHeatgDurgClimEandFromTelmConnGen2 : Outport  Port=323  OutDataTypeStr=Enum: OnOff1  PortDimensions=1
  SteerWhlHeatgPwrAct_Ngcc_SteerWhlHeatgPwrAct : Outport  Port=324  OutDataTypeStr=UInt8  PortDimensions=1
  StrtRemSts_StrtRemSts1WdStrtRemSts : Outport  Port=325  OutDataTypeStr=Enum: StrtRemSts1  PortDimensions=1
  StrtRemSts1WdStrtRemSts_Ngcc_StrtRemSts1WdStrtRemSts : Outport  Port=326  OutDataTypeStr=Enum: StrtRemSts1  PortDimensions=1
  SunCurtPosnSts_Ngcc_SunCurtPosnSts : Outport  Port=327  OutDataTypeStr=Enum: WinAndRoofAndCurtPosnTyp  PortDimensions=1
  SunRoofPosnSts_Ngcc_SunRoofPosnSts : Outport  Port=328  OutDataTypeStr=Enum: WinAndRoofAndCurtPosnTyp  PortDimensions=1
  TelmPM25Req_Ngcc_TelmPM25Req : Outport  Port=329  OutDataTypeStr=Enum: ReqSts1  PortDimensions=1
  TiDrvgCycPred_TiDrvgCycPred : Outport  Port=330  OutDataTypeStr=Ti43  PortDimensions=1
  TrSts_Ngcc_TrSts : Outport  Port=331  OutDataTypeStr=Enum: DoorSts2  PortDimensions=1
  VehModMngtGlbSafe1_Ngcc_VehModMngtGlbSafe1 : Outport  Port=332  OutDataTypeStr=Bus: VehModMngtGlbSafe1  PortDimensions=1  BusOutputAsStruct=on
  VehSpdLgt_Ngcc_VehSpdLgtSafe : Outport  Port=333  OutDataTypeStr=Bus: VehSpdLgtSafe1  PortDimensions=1  BusOutputAsStruct=on
  WadeProtnSts_Ngcc_WadeProtnSts : Outport  Port=334  OutDataTypeStr=Enum: OnOff1  PortDimensions=1
  WinGlbCmd_Ngcc_WinGlbCmd : Outport  Port=335  OutDataTypeStr=Enum: OpenClsGlbCmd  PortDimensions=1
  WinPosnStsAtDrvrReNgcc_WinPosnStsAtDrvrReNgcc : Outport  Port=336  OutDataTypeStr=Enum: WinAndRoofAndCurtPosnTyp  PortDimensions=1
  WinPosnStsAtPassReNgcc_WinPosnStsAtPassRe : Outport  Port=337  OutDataTypeStr=Enum: WinAndRoofAndCurtPosnTyp  PortDimensions=1
  WinPosnStsPass_WinPosnStsAtPass : Outport  Port=338  OutDataTypeStr=Enum: WinAndRoofAndCurtPosnTyp  PortDimensions=1
  WipgInfo_Ngcc_WipgInfo : Outport  Port=339  OutDataTypeStr=Bus: WipgInfo  PortDimensions=1  BusOutputAsStruct=on
  WtrPmpActvnReq_WtrPmpActvnReq : Outport  Port=340  OutDataTypeStr=Enum: OnOff1  PortDimensions=1
  wires:
  ActvnOfWshrFrntSafe_ActvnOfWshrFrntSafe/1 -> SdbRxSigProc_Runnable_sys/1(ActvnOfWshrFrntSafe_ActvnOfWshrFrntSafe_read)
  AirTFromHeatgEstimd_AirTFromHeatgEstimd/1 -> SdbRxSigProc_Runnable_sys/2(AirTFromHeatgEstimd_AirTFromHeatgEstimd_read)
  AmbTRaw_AmbTRaw/1 -> SdbRxSigProc_Runnable_sys/3(AmbTRaw_AmbTRaw_read)
  BltLockStAtDrvr_BltLockStAtDrvr/1 -> SdbRxSigProc_Runnable_sys/4(BltLockStAtDrvr_BltLockStAtDrvr_read)
  BltLockStAtPass_BltLockStAtPass/1 -> SdbRxSigProc_Runnable_sys/5(BltLockStAtPass_BltLockStAtPass_read)
  BltLockStAtRowSecLe_BltLockStAtRowSecLe/1 -> SdbRxSigProc_Runnable_sys/6(BltLockStAtRowSecLe_BltLockStAtRowSecLe_read)
  BltLockStAtRowSecMid_BltLockStAtRowSecMid/1 -> SdbRxSigProc_Runnable_sys/7(BltLockStAtRowSecMid_BltLockStAtRowSecMid_read)
  BltLockStAtRowSecRi_BltLockStAtRowSecRi/1 -> SdbRxSigProc_Runnable_sys/8(BltLockStAtRowSecRi_BltLockStAtRowSecRi_read)
  BltLockStAtRowThrdLe_BltLockStAtRowThrdLe/1 -> SdbRxSigProc_Runnable_sys/9(BltLockStAtRowThrdLe_BltLockStAtRowThrdLe_read)
  BltLockStAtRowThrdRi_BltLockStAtRowThrdRi/1 -> SdbRxSigProc_Runnable_sys/10(BltLockStAtRowThrdRi_BltLockStAtRowThrdRi_read)
  BookTrvlClimaActvReq_BookTrvlClimaActvReq/1 -> SdbRxSigProc_Runnable_sys/11(BookTrvlClimaActvReq_BookTrvlClimaActvReq_read)
  CamSts_CamSts/1 -> SdbRxSigProc_Runnable_sys/12(CamSts_CamSts_read)
  CarTiGlb_CarTiGlb/1 -> SdbRxSigProc_Runnable_sys/13(CarTiGlb_CarTiGlb_read)
  ClimaCfgForSnsrHumPrsnt1_ClimaCfgForSnsrHumPrsnt1/1 -> SdbRxSigProc_Runnable_sys/14(ClimaCfgForSnsrHumPrsnt1_ClimaCfgForSnsrHumPrsnt1_read)
  ClimaCmd_ClimaCmd/1 -> SdbRxSigProc_Runnable_sys/15(ClimaCmd_ClimaCmd_read)
  ClimaPwrAllwd_ClimaPwrAllwd/1 -> SdbRxSigProc_Runnable_sys/16(ClimaPwrAllwd_ClimaPwrAllwd_read)
  CmpmtInAirPmAQI_CmpmtInAirPmAQI/1 -> SdbRxSigProc_Runnable_sys/17(CmpmtInAirPmAQI_CmpmtInAirPmAQI_read)
  CmpmtInAirPmDnsty_CmpmtInAirPmDnsty/1 -> SdbRxSigProc_Runnable_sys/18(CmpmtInAirPmDnsty_CmpmtInAirPmDnsty_read)
  CmptmtCoolgSts_CmptmtCoolgSts/1 -> SdbRxSigProc_Runnable_sys/19(CmptmtCoolgSts_CmptmtCoolgSts_read)
  CmptmtFrntWindDewT_CmptmtFrntWindDewT/1 -> SdbRxSigProc_Runnable_sys/20(CmptmtFrntWindDewT_CmptmtFrntWindDewT_read)
  CmptmtFrntWindT_CmptmtFrntWindT/1 -> SdbRxSigProc_Runnable_sys/21(CmptmtFrntWindT_CmptmtFrntWindT_read)
  CmptmtRelHum_CmptmtRelHum/1 -> SdbRxSigProc_Runnable_sys/22(CmptmtRelHum_CmptmtRelHum_read)
  CoolgLoadGroup_CoolgLoadGroup/1 -> SdbRxSigProc_Runnable_sys/23(CoolgLoadGroup_CoolgLoadGroup_read)
  DefrstSts_DefrstSts/1 -> SdbRxSigProc_Runnable_sys/24(DefrstSts_DefrstSts_read)
  DoorDrvrSts_DoorDrvrSts/1 -> SdbRxSigProc_Runnable_sys/25(DoorDrvrSts_DoorDrvrSts_read)
  DoorLeReSts_DoorLeReSts/1 -> SdbRxSigProc_Runnable_sys/26(DoorLeReSts_DoorLeReSts_read)
  DoorPassSts_DoorPassSts/1 -> SdbRxSigProc_Runnable_sys/27(DoorPassSts_DoorPassSts_read)
  DoorRiReSts_DoorRiReSts/1 -> SdbRxSigProc_Runnable_sys/28(DoorRiReSts_DoorRiReSts_read)
  DrvModReq_DrvModReq/1 -> SdbRxSigProc_Runnable_sys/29(DrvModReq_DrvModReq_read)
  DrvrAirCdnrSetg_DrvrAirCdnrSetg/1 -> SdbRxSigProc_Runnable_sys/30(DrvrAirCdnrSetg_DrvrAirCdnrSetg_read)
  DrvrSeatHeatgActlPwrCns_DrvrSeatHeatgActlPwrCns/1 -> SdbRxSigProc_Runnable_sys/31(DrvrSeatHeatgActlPwrCns_DrvrSeatHeatgActlPwrCns_read)
  DrvrSeatHeatgDesPwrCns_DrvrSeatHeatgDesPwrCns/1 -> SdbRxSigProc_Runnable_sys/32(DrvrSeatHeatgDesPwrCns_DrvrSeatHeatgDesPwrCns_read)
  DrvrSeatVentnActlPwrCns_DrvrSeatVentnActlPwrCns/1 -> SdbRxSigProc_Runnable_sys/33(DrvrSeatVentnActlPwrCns_DrvrSeatVentnActlPwrCns_read)
  DrvrSeatVentnDesPwrCns_DrvrSeatVentnDesPwrCns/1 -> SdbRxSigProc_Runnable_sys/34(DrvrSeatVentnDesPwrCns_DrvrSeatVentnDesPwrCns_read)
  EgyCnsAllwdForClima_EgyCnsAllwdForClima/1 -> SdbRxSigProc_Runnable_sys/35(EgyCnsAllwdForClima_EgyCnsAllwdForClima_read)
  EngT_EngT/1 -> SdbRxSigProc_Runnable_sys/36(EngT_EngT_read)
  HMIClimaEgySaveReq_HMIClimaEgySaveReq/1 -> SdbRxSigProc_Runnable_sys/37(HMIClimaEgySaveReq_HMIClimaEgySaveReq_read)
  HpcDeiceReq_HpcDeiceReq/1 -> SdbRxSigProc_Runnable_sys/38(HpcDeiceReq_HpcDeiceReq_read)
  HvacFanSts2HvacFanBattI2_HvacFanSts2HvacFanBattI2/1 -> SdbRxSigProc_Runnable_sys/39(HvacFanSts2HvacFanBattI2_HvacFanSts2HvacFanBattI2_read)
  HvacFanSts2HvacFanMotU2_HvacFanSts2HvacFanMotU2/1 -> SdbRxSigProc_Runnable_sys/40(HvacFanSts2HvacFanMotU2_HvacFanSts2HvacFanMotU2_read)